simulink model "hipsr_parkes_nar"  (Simulink 7.8) (continued)
== system hipsr_parkes_nar == (continued)
      mask init:
      | pipeline_init(gcb,'latency',latency);
      settings: AttributesFormatString=Latency = %<latency>
      mask Display:
      | port_label('input', 1, 'd');
      | port_label('output', 1, 'q');
      | disp(['Z^-', num2str(latency)]);
  pipeline25 : Subsystem  AncestorBlock=casper_library_delays/pipeline  mask(pipeline){latency=1}
      mask help:
      | An explicitly laid-out delay line to avoid mapping to SRL16s
      | for use in pipelining to help achieve timing closure.
      mask init:
      | pipeline_init(gcb,'latency',latency);
      settings: AttributesFormatString=Latency = %<latency>
      mask Display:
      | port_label('input', 1, 'd');
      | port_label('output', 1, 'q');
      | disp(['Z^-', num2str(latency)]);
  pipeline26 : Subsystem  AncestorBlock=casper_library_delays/pipeline  mask(pipeline){latency=1}
      mask help:
      | An explicitly laid-out delay line to avoid mapping to SRL16s
      | for use in pipelining to help achieve timing closure.
      mask init:
      | pipeline_init(gcb,'latency',latency);
      settings: AttributesFormatString=Latency = %<latency>
      mask Display:
      | port_label('input', 1, 'd');
      | port_label('output', 1, 'q');
      | disp(['Z^-', num2str(latency)]);
  pipeline27 : Subsystem  AncestorBlock=casper_library_delays/pipeline  mask(pipeline){latency=1}
      mask help:
      | An explicitly laid-out delay line to avoid mapping to SRL16s
      | for use in pipelining to help achieve timing closure.
      mask init:
      | pipeline_init(gcb,'latency',latency);
      settings: AttributesFormatString=Latency = %<latency>
      mask Display:
      | port_label('input', 1, 'd');
      | port_label('output', 1, 'q');
      | disp(['Z^-', num2str(latency)]);
  pipeline28 : Subsystem  AncestorBlock=casper_library_delays/pipeline  mask(pipeline){latency=1}
      mask help:
      | An explicitly laid-out delay line to avoid mapping to SRL16s
      | for use in pipelining to help achieve timing closure.
      mask init:
      | pipeline_init(gcb,'latency',latency);
      settings: AttributesFormatString=Latency = %<latency>
      mask Display:
      | port_label('input', 1, 'd');
      | port_label('output', 1, 'q');
      | disp(['Z^-', num2str(latency)]);
  pipeline29 : Subsystem  AncestorBlock=casper_library_delays/pipeline  mask(pipeline){latency=1}
      mask help:
      | An explicitly laid-out delay line to avoid mapping to SRL16s
      | for use in pipelining to help achieve timing closure.
      mask init:
      | pipeline_init(gcb,'latency',latency);
      settings: AttributesFormatString=Latency = %<latency>
      mask Display:
      | port_label('input', 1, 'd');
      | port_label('output', 1, 'q');
      | disp(['Z^-', num2str(latency)]);
  pipeline3 : Subsystem  AncestorBlock=casper_library_delays/pipeline  mask(pipeline){latency=1}
      mask help:
      | An explicitly laid-out delay line to avoid mapping to SRL16s
      | for use in pipelining to help achieve timing closure.
      mask init:
      | pipeline_init(gcb,'latency',latency);
      settings: AttributesFormatString=Latency = %<latency>
      mask Display:
      | port_label('input', 1, 'd');
      | port_label('output', 1, 'q');
      | disp(['Z^-', num2str(latency)]);
  pipeline30 : Subsystem  AncestorBlock=casper_library_delays/pipeline  mask(pipeline){latency=1}
      mask help:
      | An explicitly laid-out delay line to avoid mapping to SRL16s
      | for use in pipelining to help achieve timing closure.
      mask init:
      | pipeline_init(gcb,'latency',latency);
      settings: AttributesFormatString=Latency = %<latency>
      mask Display:
      | port_label('input', 1, 'd');
      | port_label('output', 1, 'q');
      | disp(['Z^-', num2str(latency)]);
  pipeline32 : Subsystem  AncestorBlock=casper_library_delays/pipeline  mask(pipeline){latency=1}
      mask help:
      | An explicitly laid-out delay line to avoid mapping to SRL16s
      | for use in pipelining to help achieve timing closure.
      mask init:
      | pipeline_init(gcb,'latency',latency);
      settings: AttributesFormatString=Latency = %<latency>
      mask Display:
      | port_label('input', 1, 'd');
      | port_label('output', 1, 'q');
      | disp(['Z^-', num2str(latency)]);
  pipeline33 : Subsystem  AncestorBlock=casper_library_delays/pipeline  mask(pipeline){latency=1}
      mask help:
      | An explicitly laid-out delay line to avoid mapping to SRL16s
      | for use in pipelining to help achieve timing closure.
      mask init:
      | pipeline_init(gcb,'latency',latency);
      settings: AttributesFormatString=Latency = %<latency>
      mask Display:
      | port_label('input', 1, 'd');
      | port_label('output', 1, 'q');
      | disp(['Z^-', num2str(latency)]);
  pipeline34 : Subsystem  AncestorBlock=casper_library_delays/pipeline  mask(pipeline){latency=1}
      mask help:
      | An explicitly laid-out delay line to avoid mapping to SRL16s
      | for use in pipelining to help achieve timing closure.
      mask init:
      | pipeline_init(gcb,'latency',latency);
      settings: AttributesFormatString=Latency = %<latency>
      mask Display:
      | port_label('input', 1, 'd');
      | port_label('output', 1, 'q');
      | disp(['Z^-', num2str(latency)]);
  pipeline35 : Subsystem  AncestorBlock=casper_library_delays/pipeline  mask(pipeline){latency=1}
      mask help:
      | An explicitly laid-out delay line to avoid mapping to SRL16s
      | for use in pipelining to help achieve timing closure.
      mask init:
      | pipeline_init(gcb,'latency',latency);
      settings: AttributesFormatString=Latency = %<latency>
      mask Display:
      | port_label('input', 1, 'd');
      | port_label('output', 1, 'q');
      | disp(['Z^-', num2str(latency)]);
  pipeline36 : Subsystem  AncestorBlock=casper_library_delays/pipeline  mask(pipeline){latency=1}
      mask help:
      | An explicitly laid-out delay line to avoid mapping to SRL16s
      | for use in pipelining to help achieve timing closure.
      mask init:
      | pipeline_init(gcb,'latency',latency);
      settings: AttributesFormatString=Latency = %<latency>
      mask Display:
      | port_label('input', 1, 'd');
      | port_label('output', 1, 'q');
      | disp(['Z^-', num2str(latency)]);
  pipeline37 : Subsystem  AncestorBlock=casper_library_delays/pipeline  mask(pipeline){latency=1}
      mask help:
      | An explicitly laid-out delay line to avoid mapping to SRL16s
      | for use in pipelining to help achieve timing closure.
      mask init:
      | pipeline_init(gcb,'latency',latency);
      settings: AttributesFormatString=Latency = %<latency>
      mask Display:
      | port_label('input', 1, 'd');
      | port_label('output', 1, 'q');
      | disp(['Z^-', num2str(latency)]);
  pipeline38 : Subsystem  AncestorBlock=casper_library_delays/pipeline  mask(pipeline){latency=1}
      mask help:
      | An explicitly laid-out delay line to avoid mapping to SRL16s
      | for use in pipelining to help achieve timing closure.
      mask init:
      | pipeline_init(gcb,'latency',latency);
      settings: AttributesFormatString=Latency = %<latency>
      mask Display:
      | port_label('input', 1, 'd');
      | port_label('output', 1, 'q');
      | disp(['Z^-', num2str(latency)]);
  pipeline39 : Subsystem  AncestorBlock=casper_library_delays/pipeline  mask(pipeline){latency=1}
      mask help:
      | An explicitly laid-out delay line to avoid mapping to SRL16s
      | for use in pipelining to help achieve timing closure.
      mask init:
      | pipeline_init(gcb,'latency',latency);
      settings: AttributesFormatString=Latency = %<latency>
      mask Display:
      | port_label('input', 1, 'd');
      | port_label('output', 1, 'q');
      | disp(['Z^-', num2str(latency)]);
  pipeline4 : Subsystem  AncestorBlock=casper_library_delays/pipeline  mask(pipeline){latency=1}
      mask help:
      | An explicitly laid-out delay line to avoid mapping to SRL16s
      | for use in pipelining to help achieve timing closure.
      mask init:
      | pipeline_init(gcb,'latency',latency);
      settings: AttributesFormatString=Latency = %<latency>
      mask Display:
      | port_label('input', 1, 'd');
      | port_label('output', 1, 'q');
      | disp(['Z^-', num2str(latency)]);
  pipeline40 : Subsystem  AncestorBlock=casper_library_delays/pipeline  mask(pipeline){latency=1}
      mask help:
      | An explicitly laid-out delay line to avoid mapping to SRL16s
      | for use in pipelining to help achieve timing closure.
      mask init:
      | pipeline_init(gcb,'latency',latency);
      settings: AttributesFormatString=Latency = %<latency>
      mask Display:
      | port_label('input', 1, 'd');
      | port_label('output', 1, 'q');
      | disp(['Z^-', num2str(latency)]);
  pipeline41 : Subsystem  AncestorBlock=casper_library_delays/pipeline  mask(pipeline){latency=1}
      mask help:
      | An explicitly laid-out delay line to avoid mapping to SRL16s
      | for use in pipelining to help achieve timing closure.
      mask init:
      | pipeline_init(gcb,'latency',latency);
      settings: AttributesFormatString=Latency = %<latency>
      mask Display:
      | port_label('input', 1, 'd');
      | port_label('output', 1, 'q');
      | disp(['Z^-', num2str(latency)]);
  pipeline42 : Subsystem  AncestorBlock=casper_library_delays/pipeline  mask(pipeline){latency=1}
      mask help:
      | An explicitly laid-out delay line to avoid mapping to SRL16s
      | for use in pipelining to help achieve timing closure.
      mask init:
      | pipeline_init(gcb,'latency',latency);
      settings: AttributesFormatString=Latency = %<latency>
      mask Display:
      | port_label('input', 1, 'd');
      | port_label('output', 1, 'q');
      | disp(['Z^-', num2str(latency)]);
  pipeline43 : Subsystem  AncestorBlock=casper_library_delays/pipeline  mask(pipeline){latency=1}
      mask help:
      | An explicitly laid-out delay line to avoid mapping to SRL16s
      | for use in pipelining to help achieve timing closure.
      mask init:
      | pipeline_init(gcb,'latency',latency);
      settings: AttributesFormatString=Latency = %<latency>
      mask Display:
      | port_label('input', 1, 'd');
      | port_label('output', 1, 'q');
      | disp(['Z^-', num2str(latency)]);
  pipeline44 : Subsystem  AncestorBlock=casper_library_delays/pipeline  mask(pipeline){latency=1}
      mask help:
      | An explicitly laid-out delay line to avoid mapping to SRL16s
      | for use in pipelining to help achieve timing closure.
      mask init:
      | pipeline_init(gcb,'latency',latency);
      settings: AttributesFormatString=Latency = %<latency>
      mask Display:
      | port_label('input', 1, 'd');
      | port_label('output', 1, 'q');
      | disp(['Z^-', num2str(latency)]);
  pipeline45 : Subsystem  AncestorBlock=casper_library_delays/pipeline  mask(pipeline){latency=1}
      mask help:
      | An explicitly laid-out delay line to avoid mapping to SRL16s
      | for use in pipelining to help achieve timing closure.
      mask init:
      | pipeline_init(gcb,'latency',latency);
      settings: AttributesFormatString=Latency = %<latency>
      mask Display:
      | port_label('input', 1, 'd');
      | port_label('output', 1, 'q');
      | disp(['Z^-', num2str(latency)]);
  pipeline46 : Subsystem  AncestorBlock=casper_library_delays/pipeline  mask(pipeline){latency=1}
      mask help:
      | An explicitly laid-out delay line to avoid mapping to SRL16s
      | for use in pipelining to help achieve timing closure.
      mask init:
      | pipeline_init(gcb,'latency',latency);
      settings: AttributesFormatString=Latency = %<latency>
      mask Display:
      | port_label('input', 1, 'd');
      | port_label('output', 1, 'q');
      | disp(['Z^-', num2str(latency)]);
  pipeline47 : Subsystem  AncestorBlock=casper_library_delays/pipeline  mask(pipeline){latency=1}
      mask help:
      | An explicitly laid-out delay line to avoid mapping to SRL16s
      | for use in pipelining to help achieve timing closure.
      mask init:
      | pipeline_init(gcb,'latency',latency);
      settings: AttributesFormatString=Latency = %<latency>
      mask Display:
      | port_label('input', 1, 'd');
      | port_label('output', 1, 'q');
      | disp(['Z^-', num2str(latency)]);
  pipeline48 : Subsystem  AncestorBlock=casper_library_delays/pipeline  mask(pipeline){latency=1}
      mask help:
      | An explicitly laid-out delay line to avoid mapping to SRL16s
      | for use in pipelining to help achieve timing closure.
      mask init:
      | pipeline_init(gcb,'latency',latency);
      settings: AttributesFormatString=Latency = %<latency>
      mask Display:
      | port_label('input', 1, 'd');
      | port_label('output', 1, 'q');
      | disp(['Z^-', num2str(latency)]);
  pipeline49 : Subsystem  AncestorBlock=casper_library_delays/pipeline  mask(pipeline){latency=1}
      mask help:
      | An explicitly laid-out delay line to avoid mapping to SRL16s
      | for use in pipelining to help achieve timing closure.
      mask init:
      | pipeline_init(gcb,'latency',latency);
      settings: AttributesFormatString=Latency = %<latency>
      mask Display:
      | port_label('input', 1, 'd');
      | port_label('output', 1, 'q');
      | disp(['Z^-', num2str(latency)]);
  pipeline5 : Subsystem  AncestorBlock=casper_library_delays/pipeline  mask(pipeline){latency=1}
      mask help:
      | An explicitly laid-out delay line to avoid mapping to SRL16s
      | for use in pipelining to help achieve timing closure.
      mask init:
      | pipeline_init(gcb,'latency',latency);
      settings: AttributesFormatString=Latency = %<latency>
      mask Display:
      | port_label('input', 1, 'd');
      | port_label('output', 1, 'q');
      | disp(['Z^-', num2str(latency)]);
  pipeline50 : Subsystem  AncestorBlock=casper_library_delays/pipeline  mask(pipeline){latency=1}
      mask help:
      | An explicitly laid-out delay line to avoid mapping to SRL16s
      | for use in pipelining to help achieve timing closure.
      mask init:
      | pipeline_init(gcb,'latency',latency);
      settings: AttributesFormatString=Latency = %<latency>
      mask Display:
      | port_label('input', 1, 'd');
      | port_label('output', 1, 'q');
      | disp(['Z^-', num2str(latency)]);
  pipeline51 : Subsystem  AncestorBlock=casper_library_delays/pipeline  mask(pipeline){latency=1}
      mask help:
      | An explicitly laid-out delay line to avoid mapping to SRL16s
      | for use in pipelining to help achieve timing closure.
      mask init:
      | pipeline_init(gcb,'latency',latency);
      settings: AttributesFormatString=Latency = %<latency>
      mask Display:
      | port_label('input', 1, 'd');
      | port_label('output', 1, 'q');
      | disp(['Z^-', num2str(latency)]);
  pipeline52 : Subsystem  AncestorBlock=casper_library_delays/pipeline  mask(pipeline){latency=1}
      mask help:
      | An explicitly laid-out delay line to avoid mapping to SRL16s
      | for use in pipelining to help achieve timing closure.
      mask init:
      | pipeline_init(gcb,'latency',latency);
      settings: AttributesFormatString=Latency = %<latency>
      mask Display:
      | port_label('input', 1, 'd');
      | port_label('output', 1, 'q');
      | disp(['Z^-', num2str(latency)]);
  pipeline53 : Subsystem  AncestorBlock=casper_library_delays/pipeline  mask(pipeline){latency=1}
      mask help:
      | An explicitly laid-out delay line to avoid mapping to SRL16s
      | for use in pipelining to help achieve timing closure.
      mask init:
      | pipeline_init(gcb,'latency',latency);
      settings: AttributesFormatString=Latency = %<latency>
      mask Display:
      | port_label('input', 1, 'd');
      | port_label('output', 1, 'q');
      | disp(['Z^-', num2str(latency)]);
  pipeline6 : Subsystem  AncestorBlock=casper_library_delays/pipeline  mask(pipeline){latency=1}
      mask help:
      | An explicitly laid-out delay line to avoid mapping to SRL16s
      | for use in pipelining to help achieve timing closure.
      mask init:
      | pipeline_init(gcb,'latency',latency);
      settings: AttributesFormatString=Latency = %<latency>
      mask Display:
      | port_label('input', 1, 'd');
      | port_label('output', 1, 'q');
      | disp(['Z^-', num2str(latency)]);
  pipeline60 : Subsystem  AncestorBlock=casper_library_delays/pipeline  mask(pipeline){latency=1}
      mask help:
      | An explicitly laid-out delay line to avoid mapping to SRL16s
      | for use in pipelining to help achieve timing closure.
      mask init:
      | pipeline_init(gcb,'latency',latency);
      settings: AttributesFormatString=Latency = %<latency>
      mask Display:
      | port_label('input', 1, 'd');
      | port_label('output', 1, 'q');
      | disp(['Z^-', num2str(latency)]);
  pipeline7 : Subsystem  AncestorBlock=casper_library_delays/pipeline  mask(pipeline){latency=1}
      mask help:
      | An explicitly laid-out delay line to avoid mapping to SRL16s
      | for use in pipelining to help achieve timing closure.
      mask init:
      | pipeline_init(gcb,'latency',latency);
      settings: AttributesFormatString=Latency = %<latency>
      mask Display:
      | port_label('input', 1, 'd');
      | port_label('output', 1, 'q');
      | disp(['Z^-', num2str(latency)]);
  pipeline8 : Subsystem  AncestorBlock=casper_library_delays/pipeline  mask(pipeline){latency=1}
      mask help:
      | An explicitly laid-out delay line to avoid mapping to SRL16s
      | for use in pipelining to help achieve timing closure.
      mask init:
      | pipeline_init(gcb,'latency',latency);
      settings: AttributesFormatString=Latency = %<latency>
      mask Display:
      | port_label('input', 1, 'd');
      | port_label('output', 1, 'q');
      | disp(['Z^-', num2str(latency)]);
  pipeline9 : Subsystem  AncestorBlock=casper_library_delays/pipeline  mask(pipeline){latency=1}
      mask help:
      | An explicitly laid-out delay line to avoid mapping to SRL16s
      | for use in pipelining to help achieve timing closure.
      mask init:
      | pipeline_init(gcb,'latency',latency);
      settings: AttributesFormatString=Latency = %<latency>
      mask Display:
      | port_label('input', 1, 'd');
      | port_label('output', 1, 'q');
      | disp(['Z^-', num2str(latency)]);
  pl : Subsystem
  posedge{,1..3} (4 alike) : casper_library_misc/posedge  Tag=Rising Edge Detector
  pps_cntr : xbsIndex_r4/Counter  cnt_type=Free Running  cnt_to=Inf  operation=Up  start_count=0  cnt_by_val=1  arith_type=Unsigned  n_bits=16  bin_pt=0  load_pin=off  rst=on  en=on  explicit_period=on  period=1  dbl_ovrd=off  use_behavioral_HDL=off  implementation=Fabric  use_rpm=off  block_type=counter
  pulse_ext : casper_library_misc/pulse_ext  pulse_len=100000
  pulse_ext1 : casper_library_misc/pulse_ext  pulse_len=2^24
  pulse_ext2 : casper_library_misc/pulse_ext  pulse_len=2^24
  pulse_ext3 : casper_library_misc/pulse_ext  pulse_len=100000
  pulse_ext4 : casper_library_misc/pulse_ext  pulse_len=2^24
  quant : Subsystem  mask{bits_in=36, bits_out=18, z_cast=4, z_mult=6, quantization=Round  (unbiased: +/- Inf)}
      mask prompts: bits_in "Input bitwidth"; bits_out "Output bitwidth"; z_cast "Convert latency"; z_mult "Multiply latency"; quantization "Quantization Behaviour"
      mask help:
      | Requantizes a signal down to a desired bit width.
      | Best used in conjunction with the Stokes block.
      | Note: in_xx and in_yy are treated as unsigned, xy are treated as signed.
      mask StyleString: edit,edit,edit,edit,popup(Truncate|Round  (unbiased: +/- Inf)|Round  (unbiased: Even Values))
  snap : Subsystem
  snap_mux_x : Subsystem  AncestorBlock=casper_library_scopes/snap  mask(snap){nsamples=10}
      mask prompts: nsamples "No. of samples(2^?)"
      mask help:
      | The <i>snap</i> block provides a packaged solution to capturing data from
      | the FPGA fabric and making it accessible from the CPU.  The block comes in
      | 2 flavors: <i>snap</i> (which captures to a 32 bit wide Shared BRAM), and
      | <i>snap64</i> (which captures to 2x32 bit wide Shared BRAMs to effect a
      | 64 bit capture).
      mask Help: eval('xlWeb(''http://casper.berkeley.edu/wiki/Snap'')')
  snap_quant_xx : Subsystem  AncestorBlock=casper_library_scopes/snap  mask(snap){nsamples=10}
      mask prompts: nsamples "No. of samples(2^?)"
      mask help:
      | The <i>snap</i> block provides a packaged solution to capturing data from
      | the FPGA fabric and making it accessible from the CPU.  The block comes in
      | 2 flavors: <i>snap</i> (which captures to a 32 bit wide Shared BRAM), and
      | <i>snap64</i> (which captures to 2x32 bit wide Shared BRAMs to effect a
      | 64 bit capture).
      mask Help: eval('xlWeb(''http://casper.berkeley.edu/wiki/Snap'')')
  stokes : Subsystem
      mask Description:
      | Computes autocorrelations XX, YY and cross correlation XY.
      | Outputs XX, YY, real(XY) and imag(XY).
      | 
      | All multiplies done in DSP48Es. Inputs should be 18 bits
      | (i.e. 18 real + 18 imag = 36 bits total)
  sync : hipsr_library/sync
  uncram : Subsystem  AncestorBlock=gavrt_library/uncram  mask(uncram){num_slice=4, slice_width=32, bin_pt=0, arith_type=0}
      mask prompts: num_slice "Number of slices"; slice_width "Slice Width"; bin_pt "Output Binary Point"; arith_type "Output Arithmetic Type (0=Unsigned, 1=Signed)"
      mask help: Takes a concatenated input and slices it up into even pieces and reinterprets them as signed fixed point numbers with a given binary point.
      mask init:
      | uncram_init(gcb, ...
      |     'num_slice', num_slice, ...
      |     'slice_width', slice_width, ...
      |     'bin_pt', bin_pt, ...
      |     'arith_type', arith_type);
      settings: AttributesFormatString=UFix_32_0
  uncram1 : Subsystem  AncestorBlock=gavrt_library/uncram  mask(uncram){num_slice=4, slice_width=32, bin_pt=0, arith_type=0}
      mask prompts: num_slice "Number of slices"; slice_width "Slice Width"; bin_pt "Output Binary Point"; arith_type "Output Arithmetic Type (0=Unsigned, 1=Signed)"
      mask help: Takes a concatenated input and slices it up into even pieces and reinterprets them as signed fixed point numbers with a given binary point.
      mask init:
      | uncram_init(gcb, ...
      |     'num_slice', num_slice, ...
      |     'slice_width', slice_width, ...
      |     'bin_pt', bin_pt, ...
      |     'arith_type', arith_type);
      settings: AttributesFormatString=UFix_32_0
  vacc_signed : Subsystem  AncestorBlock=gavrt_library/vacc  mask(vacc){veclen=12, ninputs=4, max_accum=18, arith_type=1, in_bit_width=18, in_bin_pt=0, out_bit_width=32, out_bin_pt=0, register_din=0, add_latency=2, bram_latency=3, mux_latency=1}
      mask prompts: veclen "Vector Length (2^?)"; ninputs "Number of Simultaneous Vectors"; max_accum "Maximum # Accumulations (2^?)"; arith_type "Arithmetic Type (0=Unsigned, 1=Signed)"; in_bit_width "Input Bit Width"; in_bin_pt "Input Binary Point"; out_bit_width "Output Bit Width"; out_bin_pt "Output Binary Point"; register_din "Register Input Data  (1 = Enable Register Retiming on input delay)"; add_latency "Add Latency"; bram_latency "BRAM Latency"; mux_latency "Mux Latency"
      mask help:
      | Sync is expected the clock before the first data to be accumulated.
      | Use the Cram block to feed din for multiple simultaneous inputs.
      | acc_len is the number of vectors to sum.
      | valid is high while a valid accumulation is being clocked out on dout.
      | If only one sync pulse is provided, valid accumulations will repeatedly appear on dout as they become available.
      | Undesired behavior may occur if acc_len is changed without a new sync pulse.
      | Note: The output is an unsigned concatenation of the output streams. Thus, even with only one stream, the output must be recast before using.
      | Register Input Data controls the "Enable Register Retiming" parameter of the delays on 'din'.
      mask init:
      | vacc_init(gcb, ...
      |     'veclen', veclen, ...
      |     'ninputs', ninputs, ...
      |     'max_accum', max_accum, ...
      |     'arith_type', arith_type, ...
      |     'in_bit_width', in_bit_width, ...
      |     'in_bin_pt', in_bin_pt, ...
      |     'out_bit_width', out_bit_width, ...
      |     'out_bin_pt', out_bin_pt, ...
      |     'register_din', register_din, ...
      |     'add_latency', add_latency, ...
      |     'bram_latency', bram_latency, ...
      |     'mux_latency', mux_latency);
      settings: AttributesFormatString=|
      | vector length=4096, inputs=4
      | max accumulations=2^18
  vacc_unsigned : Subsystem  AncestorBlock=gavrt_library/vacc  mask(vacc){veclen=12, ninputs=4, max_accum=18, arith_type=0, in_bit_width=18, in_bin_pt=0, out_bit_width=32, out_bin_pt=0, register_din=0, add_latency=2, bram_latency=3, mux_latency=1}
      mask prompts: veclen "Vector Length (2^?)"; ninputs "Number of Simultaneous Vectors"; max_accum "Maximum # Accumulations (2^?)"; arith_type "Arithmetic Type (0=Unsigned, 1=Signed)"; in_bit_width "Input Bit Width"; in_bin_pt "Input Binary Point"; out_bit_width "Output Bit Width"; out_bin_pt "Output Binary Point"; register_din "Register Input Data  (1 = Enable Register Retiming on input delay)"; add_latency "Add Latency"; bram_latency "BRAM Latency"; mux_latency "Mux Latency"
      mask help:
      | Sync is expected the clock before the first data to be accumulated.
      | Use the Cram block to feed din for multiple simultaneous inputs.
      | acc_len is the number of vectors to sum.
      | valid is high while a valid accumulation is being clocked out on dout.
      | If only one sync pulse is provided, valid accumulations will repeatedly appear on dout as they become available.
      | Undesired behavior may occur if acc_len is changed without a new sync pulse.
      | Note: The output is an unsigned concatenation of the output streams. Thus, even with only one stream, the output must be recast before using.
      | Register Input Data controls the "Enable Register Retiming" parameter of the delays on 'din'.
      mask init: same as hipsr_parkes_nar/vacc_signed
      settings: AttributesFormatString=|
      | vector length=4096, inputs=4
      | max accumulations=2^18
  wires:
  Band-Limited White Noise/1 -> adc0/1, adc0/2
  Pulse Generator/1 -> adc0/3
  Constant/1 -> adc0/4
  [sync_gen] -> pfb1/1
  adc0/9 -> or0/1
  adc0/10 -> or0/2
  adc0/11 -> or0/3
  adc0/12 -> or0/4
  adc0/13 -> or1/1
  adc0/14 -> or1/2
  adc0/15 -> or1/3
  adc0/16 -> or1/4
  adc0/17 -> Arnie9/1
  cram/1(Out) -> vacc_unsigned/2(din)
  [acc_len] -> vacc_unsigned/3(acc_len)
  vacc_unsigned/2(dout) -> uncram/1(In)
  master_reset/1 -> pipeline51/1(d)
  Constant19/1 -> master_reset/1
  Constant16/1 -> acc_len/1
  acc_len/1 -> pipeline60/1(d)
  pipeline51/1(q) -> Slice2/1
  Slice2/1 -> posedge3/1
  pipeline60/1(q) -> [acc_len]
  Constant3/1 -> fft_shift/1
  fft_shift/1 -> pipeline49/1(d)
  pipeline49/1(q) -> [fft_shift]
  pipeline43/1(q) -> o_fft_of/1
  o_fft_of/1 -> Terminator3/1
  pipeline44/1(q) -> o_adc0_clip/1
  [adc0_clip] -> pulse_ext3/1
  o_adc0_clip/1 -> Terminator15/1
  acc_cntr/1 -> pipeline45/1(d)
  o_acc_cnt/1 -> Terminator2/1
  pipeline45/1(q) -> o_acc_cnt/1
  [cnt_rst] -> acc_cntr/1
  Delay22/1 -> acc_cntr/2
  [new_acc] -> Delay22/1
  mux_sel/1 -> pipeline50/1(d)
  Constant7/1 -> mux_sel/1
  Slice3/1 -> [mux_sel]
  [mux_sel] -> adc_mux/1(mux_sel)
  [adc0_i0] -> adc_mux/2(i0)
  [adc0_i1] -> adc_mux/3(i1)
  [adc0_i2] -> adc_mux/4(i2)
  [adc0_i3] -> adc_mux/5(i3)
  [mux_sel] -> adc_mux1/1(mux_sel)
  [adc0_q0] -> adc_mux1/2(i0)
  [adc0_q1] -> adc_mux1/3(i1)
  [adc0_q2] -> adc_mux1/4(i2)
  [adc0_q3] -> adc_mux1/5(i3)
  adc_mux/1(Out0) -> [mux_i0]
  adc_mux/2(Out2) -> [mux_i1]
  adc_mux/3(Out3) -> [mux_i2]
  adc_mux/4(Out4) -> [mux_i3]
  [cnt_rst] -> noise_source/1
  noise_source/1 -> Reinterpret/1
  noise_source/2 -> Reinterpret1/1
  noise_source/3 -> Reinterpret2/1
  noise_source/4 -> Reinterpret3/1
  noise_source/5 -> Reinterpret4/1
  noise_source/6 -> Reinterpret5/1
  noise_source/7 -> Reinterpret6/1
  noise_source/8 -> Reinterpret7/1
  [m0] -> adc_mux1/6(n0)
  [m1] -> adc_mux1/7(n1)
  [m2] -> adc_mux1/8(n2)
  [m3] -> adc_mux1/9(n3)
  or0/1 -> [adc0_clip]
  Constant6/1 -> snap_mux_x/3(we), snap_mux_x/2(trig)
  cram1/1 -> snap_mux_x/1(din)
  [mux_i0] -> cram1/1
  [mux_i1] -> cram1/2
  [mux_i2] -> cram1/3
  [mux_i3] -> cram1/4
  posedge/1 -> [new_acc], snap/1(trig)
  pipeline2/1(q) -> snap/2(we)
  Reinterpret/1 -> Arnie1/1
  Reinterpret1/1 -> Arnie2/1
  Reinterpret2/1 -> Arnie3/1
  Reinterpret3/1 -> Arnie4/1
  Reinterpret4/1 -> [m0]
  Reinterpret5/1 -> [m1]
  Reinterpret6/1 -> [m2]
  Reinterpret7/1 -> [m3]
  pipeline5/1(q) -> snap/5(in_xx0)
  pipeline6/1(q) -> snap/6(in_yy0)
  pipeline7/1(q) -> snap/7(in_re_xy0)
  pipeline8/1(q) -> snap/8(in_im_xy0)
  [mux_i0] -> pfb1/2
  [mux_i1] -> pfb1/3
  [mux_i2] -> pfb1/4
  [mux_i3] -> pfb1/5
  adc_mux1/1(Out0) -> [mux_q0]
  adc_mux1/2(Out2) -> [mux_q1]
  adc_mux1/3(Out3) -> [mux_q2]
  adc_mux1/4(Out4) -> [mux_q3]
  [mux_q0] -> pfb1/6
  [mux_q1] -> pfb1/7
  [mux_q2] -> pfb1/8
  [mux_q3] -> pfb1/9
  pipeline28/1(q) -> vacc_unsigned/1(sync)
  pipeline29/1(q) -> cram/1(In1)
  pipeline30/1(q) -> cram/2(In2)
  pulse_ext3/1 -> pipeline44/1(d)
  [fft_of] -> pulse_ext/1
  pulse_ext/1 -> pipeline43/1(d)
  [m0] -> adc_mux/6(n0)
  [m1] -> adc_mux/7(n1)
  [m2] -> adc_mux/8(n2)
  [m3] -> adc_mux/9(n3)
  cram3/1(Out) -> vacc_signed/2(din)
  [acc_len] -> vacc_signed/3(acc_len)
  vacc_signed/2(dout) -> uncram1/1(In)
  Constant1/1 -> pipeline47/1(d)
  pipeline47/1(q) -> gpio_b_oe/1
  gpio_b_oe/1 -> Terminator1/1
  pipeline52/1(q) -> gpio_b_led1/1
  gpio_b_led1/1 -> Terminator5/1
  [new_acc] -> pulse_ext1/1
  pulse_ext1/1 -> pipeline52/1(d)
  or1/1 -> [pps]
  [pps] -> sync/2
  sync/1 -> [sync_gen]
  pps_cntr/1 -> pipeline46/1(d)
  pipeline46/1(q) -> o_pps_cnt/1
  o_pps_cnt/1 -> Terminator6/1
  [cnt_rst] -> pps_cntr/1
  posedge1/1 -> pps_cntr/2
  [pps] -> posedge1/1
  posedge3/1 -> [cnt_rst]
  [cnt_rst] -> sync/1
  pipeline48/1(q) -> gpio_b_led0/1
  gpio_b_led0/1 -> Terminator8/1
  [pps] -> pulse_ext2/1
  pulse_ext2/1 -> pipeline48/1(d)
  pfb1/1 -> pipeline10/1(d)
  pfb1/2 -> pipeline11/1(d)
  pfb1/3 -> pipeline12/1(d)
  pfb1/4 -> pipeline13/1(d)
  pfb1/5 -> pipeline14/1(d)
  pfb1/6 -> pipeline15/1(d)
  pfb1/7 -> pipeline16/1(d)
  pfb1/8 -> pipeline17/1(d)
  pfb1/9 -> pipeline18/1(d)
  [pfb_sync] -> fft0/1
  [fft_shift] -> fft0/2
  [ant0_out0] -> fft0/3
  [ant0_out1] -> fft0/4
  [ant0_out2] -> fft0/5
  [ant0_out3] -> fft0/6
  fft0/1 -> pipeline19/1(d)
  fft0/2 -> pipeline20/1(d)
  fft0/3 -> pipeline21/1(d)
  [pfb_sync] -> fft1/1
  [fft_shift] -> fft1/2
  [ant1_out0] -> fft1/3
  [ant1_out1] -> fft1/4
  [ant1_out2] -> fft1/5
  [ant1_out3] -> fft1/6
  fft1/1 -> pipeline23/1(d)
  fft1/2 -> pipeline24/1(d)
  fft1/3 -> pipeline25/1(d)
  [fft_sync_out] -> stokes/1(sync)
  [fft_out0] -> stokes/3(in_x0)
  [fft_out1] -> stokes/4(in_x1)
  [fft1_out0] -> stokes/5(in_y0)
  [fft1_out1] -> stokes/6(in_y1)
  stokes/1(sync_out) -> quant/1(sync)
  stokes/3(out_xx0) -> quant/3(in_xx0)
  stokes/4(out_yy0) -> quant/4(in_yy0)
  stokes/5(out_re_xy0) -> quant/5(in_re_xy)
  stokes/6(out_im_xy0) -> quant/6(in_im_xy)
  stokes/7(out_xx1) -> quant/7(in_xx1)
  stokes/8(out_yy1) -> quant/8(in_yy1)
  stokes/9(out_re_xy1) -> quant/9(in_re_xy1)
  stokes/10(out_im_xy1) -> quant/10(in_im_xy1)
  pipeline32/1(q) -> cram/3(In3)
  pipeline33/1(q) -> cram/4(In4)
  quant/3(out_xx) -> [quant_xx0], Reinterpret8/1
  quant/4(out_yy) -> [quant_yy0]
  quant/5(out_re_xy) -> [quant_re_xy0]
  quant/6(out_im_xy) -> [quant_im_xy0]
  pipeline35/1(q) -> cram3/1(In1)
  pipeline36/1(q) -> cram3/2(In2)
  pipeline37/1(q) -> cram3/3(In3)
  pipeline38/1(q) -> cram3/4(In4)
  quant/7(out_xx1) -> [quant_xx1]
  quant/8(out_yy1) -> [quant_yy1]
  quant/9(out_re_xy1) -> [quant_re_xy1]
  quant/10(out_im_xy1) -> [quant_im_xy1]
  quant/1(sync_out) -> [quant_sync]
  [quant_sync] -> pipeline28/1(d)
  [quant_xx0] -> pipeline29/1(d)
  [quant_xx1] -> pipeline30/1(d)
  [quant_yy0] -> pipeline32/1(d)
  [quant_yy1] -> pipeline33/1(d)
  [quant_re_xy0] -> pipeline35/1(d)
  [quant_re_xy1] -> pipeline36/1(d)
  [quant_im_xy0] -> pipeline37/1(d)
  [quant_im_xy1] -> pipeline38/1(d)
  vacc_unsigned/1(valid) -> [vacc_valid]
  uncram/1(Out1) -> [vacc_xx0]
  uncram/2(Out2) -> [vacc_xx1]
  uncram/3(Out3) -> [vacc_yy0]
  uncram/4(Out4) -> [vacc_yy1]
  uncram1/1(Out1) -> [vacc_re_xy0]
  uncram1/2(Out2) -> [vacc_re_xy1]
  uncram1/3(Out3) -> [vacc_im_xy0]
  uncram1/4(Out4) -> [vacc_im_xy1]
  pipeline9/1(q) -> snap/9(in_xx1)
  pipeline40/1(q) -> snap/10(in_yy1)
  pipeline41/1(q) -> snap/11(in_re_xy1)
  pipeline42/1(q) -> snap/12(in_im_xy1)
  [vacc_valid] -> posedge/1, pipeline2/1(d)
  [vacc_xx0] -> pipeline5/1(d)
  [vacc_yy0] -> pipeline6/1(d)
  [vacc_re_xy0] -> pipeline7/1(d)
  [vacc_im_xy0] -> pipeline8/1(d)
  [vacc_xx1] -> pipeline9/1(d)
  [vacc_yy1] -> pipeline40/1(d)
  [vacc_re_xy1] -> pipeline41/1(d)
  [vacc_im_xy1] -> pipeline42/1(d)
  Constant4/1 -> snap_quant_xx/3(we), snap_quant_xx/2(trig)
  Convert/1 -> pipeline27/1(d)
  Reinterpret8/1 -> Convert/1
  pipeline18/1(q) -> [ant1_out3]
  pipeline17/1(q) -> [ant1_out2]
  pipeline16/1(q) -> [ant1_out1]
  pipeline15/1(q) -> [ant1_out0]
  pipeline14/1(q) -> [ant0_out3]
  pipeline13/1(q) -> [ant0_out2]
  pipeline12/1(q) -> [ant0_out1]
  pipeline11/1(q) -> [ant0_out0]
  pipeline10/1(q) -> [pfb_sync]
  fft0/4 -> pipeline22/1(d)
  fft1/4 -> pipeline26/1(d)
  [fft_sync_out1] -> stokes/2(sync1)
  stokes/2(sync_out1) -> quant/2(sync1)
  quant/2(sync_out1) -> [quant_sync1]
  [quant_sync1] -> pipeline34/1(d)
  pipeline34/1(q) -> vacc_signed/1(sync)
  vacc_signed/1(valid) -> [vacc_valid1]
  posedge2/1 -> snap/3(trig1)
  pipeline4/1(q) -> snap/4(we1)
  [vacc_valid1] -> posedge2/1, pipeline4/1(d)
  pipeline21/1(q) -> [fft_out1]
  pipeline20/1(q) -> [fft_out0]
  pipeline19/1(q) -> [fft_sync_out]
  pipeline22/1(q) -> [fft_of]
  pipeline25/1(q) -> [fft1_out1]
  pipeline24/1(q) -> [fft1_out0]
  pipeline26/1(q) -> [fft_of1]
  pipeline23/1(q) -> [fft_sync_out1]
  pipeline27/1(q) -> snap_quant_xx/1(din)
  pipeline50/1(q) -> Slice3/1
  adc0/1 -> pl/1(In1)
  adc0/2 -> pl/2(In2)
  adc0/3 -> pl/3(In3)
  adc0/4 -> pl/4(In4)
  adc0/5 -> pl/5(In5)
  adc0/6 -> pl/6(In6)
  adc0/7 -> pl/7(In7)
  adc0/8 -> pl/8(In8)
  pl/1(Out1) -> [adc0_i0]
  pl/2(Out2) -> [adc0_i1]
  pl/3(Out3) -> [adc0_i2]
  pl/4(Out4) -> [adc0_i3]
  pl/5(Out5) -> [adc0_q0]
  pl/6(Out6) -> [adc0_q1]
  pl/7(Out7) -> [adc0_q2]
  pl/8(Out8) -> [adc0_q3]
  pipeline39/1(q) -> adc_clip_led/1
  adc_clip_led/1 -> Terminator4/1
  [adc0_clip] -> pulse_ext4/1
  pulse_ext4/1 -> pipeline39/1(d)
  [sync_gen] -> nar/1(sync_in)
  [adc0_i0] -> nar/2(ant0_in0)
  [adc0_i1] -> nar/3(ant0_in1)
  [adc0_i2] -> nar/4(ant0_in2)
  [adc0_i3] -> nar/5(ant0_in3)
  [adc0_q0] -> nar/6(ant1_in0)
  [adc0_q1] -> nar/7(ant1_in1)
  [adc0_q2] -> nar/8(ant1_in2)
  [adc0_q3] -> nar/9(ant1_in3)
  nar/1(sq_wave_out) -> [sq_wave]
  pipeline1/1(q) -> gpio_a_sma/1
  gpio_a_sma/1 -> Terminator17/1
  [sq_wave] -> pipeline1/1(d)
  pipeline3/1(q) -> gpio_a_sma2/1
  gpio_a_sma2/1 -> Terminator18/1
  [sq_wave] -> pipeline3/1(d)
  Constant11/1 -> pipeline53/1(d)
  pipeline53/1(q) -> gpio_a_oe/1
  gpio_a_oe/1 -> Terminator19/1
